simulink model "TosAeb"  (R2021a)
settings: Solver=FixedStepDiscrete  StartTime=0.0  StopTime=inf  FixedStep=0.01  RelTol=1e-3
undefined symbols (not defined in the file or in its folder): ACCModSts, AccSts1, AEB_ObjectMovingOrientation, AEB_ObjectMovingState, CN_BTCActive, CN_CurrentGearR, CN_CurrentGearR_DHT, CN_PTCActive, CN_VDCActive, CN_VSC_EspOff, CN_VSC_FCW_AEB_Error, CN_VSC_FCW_AEB_NoDispay, CN_VSC_FCW_AEB_PerfmDgdtn, CN_VSC_FCW_AEB_TempUnAvlbl, CN_VSC_VehStandstill, CNaccelAvoidMax, CNaccelAvoidMin, CNbrakePedalPress, CNcomfortStrLeftAccelMax, CNcomfortStrLeftAccelMin, CNcomfortStrRightAccelMax, CNcomfortStrRightAccelMin, CNDrvDoorOpen, CNDrvModeEco, CNDrvModeNorm, CNDrvModeOther, CNDrvModeSport, CNDrvSBRreminder, CNNull, CNobjInvalidID, CNPassDoorOpen, CNPassSBRreminder, CNPEBSAccelNegInf, CNPEBSAccelNull, CNPEBSTimeInf, CNPEBSTimeNull, CNSTOPMAX, CNSTOPNULL, CNsubjWidth, CNSwtOFF, CNSwtON, CNTTCMAX, CNTTCNULL, CNVehConfigAEBCycNone, CNVehConfigAEBNone, CNVehConfigAEBPedNone, CNVehConfigFCWNone, CNVehConfigJANone, CollisionSeverity, FALSE, FCWSnvty, JACycObjCalcInfoDefaultParams, JAPedObjCalcInfoDefaultParams, JAVehObjCalcInfoDefaultParams, latCycObjCalcInfoDefaultParams, latPedObjCalcInfoDefaultParams, longObjCalcInfoDefaultParams, ModSts, movingStatus, ObjectType ... (+1112)
libraries: simulink x3031, AEBSFunctionLib x50, Ta_Library x33, AEBSStateMachineLib x23, Mylibrary x12, simulink_extras x7, ObjSelect_lib x3, dspstat3 x2
(part 30 of 102)

== system TosAeb/FcwAebJa_Step_sys/ALG_AEBSystem/ALG_AEBController/AEB_LatObjInfoCalcApp/LatPedObjCalc/LatPedCollisonAssessInfo/ObjIsInvalidJudge ==
  ObjectID : Inport
  Compare To Constant : simulink/Logic and Bit Operations/Compare To Constant  relop===  const=CNobjInvalidID  OutDataTypeStr=boolean
  ObjIsInvalid : Outport
  wires:
  ObjectID/1 -> Compare To Constant/1
  Compare To Constant/1 -> ObjIsInvalid/1

== system TosAeb/FcwAebJa_Step_sys/ALG_AEBSystem/ALG_AEBController/AEB_LatObjInfoCalcApp/LatPedObjCalc/LatPedObjCalc/ObjPreSelectCondCalc ==
  latPedObjInfo : Inport  OutDataTypeStr=Bus: BusAEBLatObj  PortDimensions=1  BusOutputAsStruct=on
  subjVel : Inport  Port=2
  Bus Selector : BusSelector  OutputSignals=ObjectID,dx,dy,ObjectExistProb,dyVariance,ObjectMovingOrientation,ObjectType,ObjectTypeCameraConfirmed,vx
  If : If  IfExpression=u1
  Merge : Merge
  ObjIsInvalidJudge : Subsystem
  TgtPreSelect : Subsystem
  TgtPreSelectDefault : Subsystem  TreatAsAtomicUnit=on
  ObjPreSelectCond : Outport
  wires:
  Merge/1 -> ObjPreSelectCond/1
  Bus Selector/1 -> ObjIsInvalidJudge/1(ObjectID)  "<ObjectID>"
  TgtPreSelectDefault/1(ObjPreSelectCond) -> Merge/1
  TgtPreSelect/1(ObjPreSelectCond) -> Merge/2
  ObjIsInvalidJudge/1(ObjIsInvalid) -> If/1
  If/2 -> TgtPreSelect/Action
  latPedObjInfo/1 -> Bus Selector/1
  If/1 -> TgtPreSelectDefault/Action
  subjVel/1 -> TgtPreSelect/9(subjVel)
  Bus Selector/2 -> TgtPreSelect/1(dx)  "<dx>"
  Bus Selector/3 -> TgtPreSelect/2(dy)  "<dy>"
  Bus Selector/4 -> TgtPreSelect/3(ObjectExistProb)  "<ObjectExistProb>"
  Bus Selector/5 -> TgtPreSelect/4(dyVariance)  "<dyVariance>"
  Bus Selector/6 -> TgtPreSelect/5(ObjectMovingOrientation)  "<ObjectMovingOrientation>"
  Bus Selector/7 -> TgtPreSelect/6(ObjectType)  "<ObjectType>"
  Bus Selector/8 -> TgtPreSelect/7(ObjectTypeCameraConfirmed)  "<ObjectTypeCameraConfirmed>"
  Bus Selector/9 -> TgtPreSelect/8(vx)  "<vx>"

== system TosAeb/FcwAebJa_Step_sys/ALG_AEBSystem/ALG_AEBController/AEB_LatObjInfoCalcApp/LatPedObjCalc/LatPedObjCalc/latPedObjInfoCalc ==
  subjInfo : Inport
  latPedObjInfo : Inport  Port=2  OutDataTypeStr=Bus: BusAEBLatObj
  Action Port : ActionPort  ActionPortLabel=else
  Bus Creator : BusCreator  Inputs=6  OutDataTypeStr=Bus: BusLatPedObjCalcInfo  NonVirtualBus=on
      settings: InheritFromInputs=off
  Bus Creator1 : BusCreator  Inputs=5
  Bus Selector : BusSelector  OutputSignals=dx,vx,ax,vy,ObjectID,ObjectTypeCameraConfirmed,dy,FR_ObjectID,FR_Dx,FR_Dy,FR_Vy,FC_ObjectID,FC_Dx,FC_Dy,FC_Vy
  Bus Selector1 : BusSelector  OutputSignals=subjVel,subjLongAccel,subjYawRate
  ObjColiisionRiskProbCalc : Subsystem
  ObjCrossFlgCal : Subsystem
  SensorTgtInfo2AbsTgtInfoTrans : Subsystem
  Terminator : Terminator
  Terminator1 : Terminator
  latPedCalcInfo : Outport
  wires:
  ObjColiisionRiskProbCalc/2(preCollisonMaxTime) -> Bus Creator/4  "pedCollisionRiskProb_TTC"
  subjInfo/1 -> ObjCrossFlgCal/1(subjInfo), ObjColiisionRiskProbCalc/3(subjInfo), Bus Selector1/1
  Bus Selector/1 -> SensorTgtInfo2AbsTgtInfoTrans/1(dx)  "<dx>"
  Bus Selector/3 -> SensorTgtInfo2AbsTgtInfoTrans/3(ax)  "<ax>"
  [ObjAbsInfo] -> ObjCrossFlgCal/3(ObjAbsInfo)
  SensorTgtInfo2AbsTgtInfoTrans/1(dxFrontBumper) -> Bus Creator1/1  "dxFrontBumper"
  ObjColiisionRiskProbCalc/4(dyCollisionRiskProbOut) -> Terminator1/1
  Bus Selector/2 -> SensorTgtInfo2AbsTgtInfoTrans/2(vx)  "<vx>"
  Bus Selector1/1 -> SensorTgtInfo2AbsTgtInfoTrans/16(subjVel)  "<subjVel>"
  ObjColiisionRiskProbCalc/3(dxCollisionRiskProbOut) -> Terminator/1
  SensorTgtInfo2AbsTgtInfoTrans/2(vxAbsObj) -> Bus Creator1/2  "vxAbsObj"
  Bus Creator1/1 -> ObjColiisionRiskProbCalc/2(ObjAbsInfo), [ObjAbsInfo]
  SensorTgtInfo2AbsTgtInfoTrans/3(axAbsObj) -> Bus Creator1/3  "axAbsObj"
  Bus Selector1/2 -> SensorTgtInfo2AbsTgtInfoTrans/17(subjLongAccel)  "<subjLongAccel>"
  Bus Creator/1 -> latPedCalcInfo/1
  latPedObjInfo/1 -> Bus Creator/1, ObjColiisionRiskProbCalc/1(ObjInfoRaw), Bus Selector/1, ObjCrossFlgCal/2(ObjInfoRaw)  "latPedObjInfo"
  ObjCrossFlgCal/1(pedPreCollisionFlg) -> Bus Creator/5  "pedPreCollisionFlg"
  [ObjAbsInfo] -> Bus Creator/2  "pedObjAbsInfo"
  ObjColiisionRiskProbCalc/1(pedCollisionRiskProb) -> Bus Creator/3  "pedCollisionRiskProb"
  Bus Selector/4 -> SensorTgtInfo2AbsTgtInfoTrans/4(vy)  "<vy>"
  Bus Selector1/3 -> SensorTgtInfo2AbsTgtInfoTrans/18(subjYawRate)  "<subjYawRate>"
  SensorTgtInfo2AbsTgtInfoTrans/4(vyCorr) -> Bus Creator1/4  "vyCorr"
  SensorTgtInfo2AbsTgtInfoTrans/5(ObjFusQuality) -> Bus Creator1/5  "ObjFusQuality"
  Bus Selector/5 -> SensorTgtInfo2AbsTgtInfoTrans/5(ObjectID)  "<ObjectID>"
  Bus Selector/6 -> SensorTgtInfo2AbsTgtInfoTrans/6(ObjectTypeCameraConfirmed)  "<ObjectTypeCameraConfirmed>"
  Bus Selector/8 -> SensorTgtInfo2AbsTgtInfoTrans/8(FR_ObjectID)  "<FR_ObjectID>"
  Bus Selector/11 -> SensorTgtInfo2AbsTgtInfoTrans/11(FR_Vy)  "<FR_Vy>"
  Bus Selector/12 -> SensorTgtInfo2AbsTgtInfoTrans/12(FC_ObjectID)  "<FC_ObjectID>"
  Bus Selector/15 -> SensorTgtInfo2AbsTgtInfoTrans/15(FC_Vy)  "<FC_Vy>"
  Bus Selector/13 -> SensorTgtInfo2AbsTgtInfoTrans/13(FC_Dx)  "<FC_Dx>"
  Bus Selector/9 -> SensorTgtInfo2AbsTgtInfoTrans/9(FR_Dx)  "<FR_Dx>"
  Bus Selector/10 -> SensorTgtInfo2AbsTgtInfoTrans/10(FR_Dy)  "<FR_Dy>"
  Bus Selector/14 -> SensorTgtInfo2AbsTgtInfoTrans/14(FC_Dy)  "<FC_Dy>"
  Bus Selector/7 -> SensorTgtInfo2AbsTgtInfoTrans/7(dy)  "<dy>"
  ObjCrossFlgCal/2(TTC) -> Bus Creator/6  "pedPreCollisionFlg_TTC"

== system TosAeb/FcwAebJa_Step_sys/ALG_AEBSystem/ALG_AEBController/AEB_LatObjInfoCalcApp/LatPedObjCalc/LatPedObjCalc/latPedObjInfoDefault ==
  Action Port : ActionPort  ActionPortLabel=if(u1)
  LatObjCalcInfoDefaultParams : Constant  Value=latPedObjCalcInfoDefaultParams  OutDataTypeStr=Bus: BusLatPedObjCalcInfo
  latPedCalcInfo : Outport
  wires:
  LatObjCalcInfoDefaultParams/1 -> latPedCalcInfo/1

== system TosAeb/FcwAebJa_Step_sys/ALG_AEBSystem/ALG_AEBController/AEB_LatObjInfoCalcApp/LatPedObjCalc/Subsystem/ObjPreSelectCondCalc ==
  latPedObjInfo : Inport  OutDataTypeStr=Bus: BusAEBLatObj  PortDimensions=1  BusOutputAsStruct=on
  subjVel : Inport  Port=2
  Bus Selector : BusSelector  OutputSignals=ObjectID,dx,dy,ObjectExistProb,dyVariance,ObjectMovingOrientation,ObjectType,ObjectTypeCameraConfirmed,vx
  If : If  IfExpression=u1
  Merge : Merge
  ObjIsInvalidJudge : Subsystem
  TgtPreSelect : Subsystem
  TgtPreSelectDefault : Subsystem  TreatAsAtomicUnit=on
  ObjPreSelectCond : Outport
  wires:
  Merge/1 -> ObjPreSelectCond/1
  Bus Selector/1 -> ObjIsInvalidJudge/1(ObjectID)  "<ObjectID>"
  TgtPreSelectDefault/1(ObjPreSelectCond) -> Merge/1
  TgtPreSelect/1(ObjPreSelectCond) -> Merge/2
  ObjIsInvalidJudge/1(ObjIsInvalid) -> If/1
  If/2 -> TgtPreSelect/Action
  latPedObjInfo/1 -> Bus Selector/1
  If/1 -> TgtPreSelectDefault/Action
  subjVel/1 -> TgtPreSelect/9(subjVel)
  Bus Selector/2 -> TgtPreSelect/1(dx)  "<dx>"
  Bus Selector/3 -> TgtPreSelect/2(dy)  "<dy>"
  Bus Selector/4 -> TgtPreSelect/3(ObjectExistProb)  "<ObjectExistProb>"
  Bus Selector/5 -> TgtPreSelect/4(dyVariance)  "<dyVariance>"
  Bus Selector/6 -> TgtPreSelect/5(ObjectMovingOrientation)  "<ObjectMovingOrientation>"
  Bus Selector/7 -> TgtPreSelect/6(ObjectType)  "<ObjectType>"
  Bus Selector/8 -> TgtPreSelect/7(ObjectTypeCameraConfirmed)  "<ObjectTypeCameraConfirmed>"
  Bus Selector/9 -> TgtPreSelect/8(vx)  "<vx>"

== system TosAeb/FcwAebJa_Step_sys/ALG_AEBSystem/ALG_AEBController/AEB_LatObjInfoCalcApp/LatPedObjCalc/Subsystem/latPedObjInfoCalc ==
  subjInfo : Inport
  latPedObjInfo : Inport  Port=2  OutDataTypeStr=Bus: BusAEBLatObj
  Action Port : ActionPort  ActionPortLabel=else
  Bus Creator : BusCreator  Inputs=6  OutDataTypeStr=Bus: BusLatPedObjCalcInfo  NonVirtualBus=on
      settings: InheritFromInputs=off
  Bus Creator1 : BusCreator  Inputs=5
  Bus Selector : BusSelector  OutputSignals=dx,vx,ax,vy,ObjectID,ObjectTypeCameraConfirmed,dy,FR_ObjectID,FR_Dx,FR_Dy,FR_Vy,FC_ObjectID,FC_Dx,FC_Dy,FC_Vy
  Bus Selector1 : BusSelector  OutputSignals=subjVel,subjLongAccel,subjYawRate
  ObjColiisionRiskProbCalc : Subsystem
  ObjCrossFlgCal : Subsystem
  SensorTgtInfo2AbsTgtInfoTrans : Subsystem
  Terminator : Terminator
  Terminator1 : Terminator
  latPedCalcInfo : Outport
  wires:
  ObjColiisionRiskProbCalc/2(preCollisonMaxTime) -> Bus Creator/4  "pedCollisionRiskProb_TTC"
  subjInfo/1 -> ObjCrossFlgCal/1(subjInfo), ObjColiisionRiskProbCalc/3(subjInfo), Bus Selector1/1
  Bus Selector/1 -> SensorTgtInfo2AbsTgtInfoTrans/1(dx)  "<dx>"
  Bus Selector/3 -> SensorTgtInfo2AbsTgtInfoTrans/3(ax)  "<ax>"
  [ObjAbsInfo] -> ObjCrossFlgCal/3(ObjAbsInfo)
  SensorTgtInfo2AbsTgtInfoTrans/1(dxFrontBumper) -> Bus Creator1/1  "dxFrontBumper"
  ObjColiisionRiskProbCalc/4(dyCollisionRiskProbOut) -> Terminator1/1
  Bus Selector/2 -> SensorTgtInfo2AbsTgtInfoTrans/2(vx)  "<vx>"
  Bus Selector1/1 -> SensorTgtInfo2AbsTgtInfoTrans/16(subjVel)  "<subjVel>"
  ObjColiisionRiskProbCalc/3(dxCollisionRiskProbOut) -> Terminator/1
  SensorTgtInfo2AbsTgtInfoTrans/2(vxAbsObj) -> Bus Creator1/2  "vxAbsObj"
  Bus Creator1/1 -> ObjColiisionRiskProbCalc/2(ObjAbsInfo), [ObjAbsInfo]
  SensorTgtInfo2AbsTgtInfoTrans/3(axAbsObj) -> Bus Creator1/3  "axAbsObj"
  Bus Selector1/2 -> SensorTgtInfo2AbsTgtInfoTrans/17(subjLongAccel)  "<subjLongAccel>"
  Bus Creator/1 -> latPedCalcInfo/1
  latPedObjInfo/1 -> Bus Creator/1, ObjColiisionRiskProbCalc/1(ObjInfoRaw), Bus Selector/1, ObjCrossFlgCal/2(ObjInfoRaw)  "latPedObjInfo"
  ObjCrossFlgCal/1(pedPreCollisionFlg) -> Bus Creator/5  "pedPreCollisionFlg"
  [ObjAbsInfo] -> Bus Creator/2  "pedObjAbsInfo"
  ObjColiisionRiskProbCalc/1(pedCollisionRiskProb) -> Bus Creator/3  "pedCollisionRiskProb"
  Bus Selector/4 -> SensorTgtInfo2AbsTgtInfoTrans/4(vy)  "<vy>"
  Bus Selector1/3 -> SensorTgtInfo2AbsTgtInfoTrans/18(subjYawRate)  "<subjYawRate>"
  SensorTgtInfo2AbsTgtInfoTrans/4(vyCorr) -> Bus Creator1/4  "vyCorr"
  SensorTgtInfo2AbsTgtInfoTrans/5(ObjFusQuality) -> Bus Creator1/5  "ObjFusQuality"
  Bus Selector/5 -> SensorTgtInfo2AbsTgtInfoTrans/5(ObjectID)  "<ObjectID>"
  Bus Selector/6 -> SensorTgtInfo2AbsTgtInfoTrans/6(ObjectTypeCameraConfirmed)  "<ObjectTypeCameraConfirmed>"
  Bus Selector/8 -> SensorTgtInfo2AbsTgtInfoTrans/8(FR_ObjectID)  "<FR_ObjectID>"
  Bus Selector/11 -> SensorTgtInfo2AbsTgtInfoTrans/11(FR_Vy)  "<FR_Vy>"
  Bus Selector/12 -> SensorTgtInfo2AbsTgtInfoTrans/12(FC_ObjectID)  "<FC_ObjectID>"
  Bus Selector/15 -> SensorTgtInfo2AbsTgtInfoTrans/15(FC_Vy)  "<FC_Vy>"
  Bus Selector/13 -> SensorTgtInfo2AbsTgtInfoTrans/13(FC_Dx)  "<FC_Dx>"
  Bus Selector/9 -> SensorTgtInfo2AbsTgtInfoTrans/9(FR_Dx)  "<FR_Dx>"
  Bus Selector/10 -> SensorTgtInfo2AbsTgtInfoTrans/10(FR_Dy)  "<FR_Dy>"
  Bus Selector/14 -> SensorTgtInfo2AbsTgtInfoTrans/14(FC_Dy)  "<FC_Dy>"
  Bus Selector/7 -> SensorTgtInfo2AbsTgtInfoTrans/7(dy)  "<dy>"
  ObjCrossFlgCal/2(TTC) -> Bus Creator/6  "pedPreCollisionFlg_TTC"

== system TosAeb/FcwAebJa_Step_sys/ALG_AEBSystem/ALG_AEBController/AEB_LatObjInfoCalcApp/LatPedObjCalc/Subsystem/latPedObjInfoDefault ==
  Action Port : ActionPort  ActionPortLabel=if(u1)
  LatObjCalcInfoDefaultParams : Constant  Value=latPedObjCalcInfoDefaultParams  OutDataTypeStr=Bus: BusLatPedObjCalcInfo
  latPedCalcInfo : Outport
  wires:
  LatObjCalcInfoDefaultParams/1 -> latPedCalcInfo/1

== system TosAeb/FcwAebJa_Step_sys/ALG_AEBSystem/ALG_AEBController/AEB_LongTgtInfoCalc/LongObjCalcInfoCalc/AEBS_LongCollisionAssesApp/DriverReactionTimeCalc ==
  ACCState : Inport  OutDataTypeStr=Enum: ACCModSts
  BrakePedalStatus_G : Inport  Port=2
  ESP_MasterCylindBrakePress : Inport  Port=3
  AccrPedlPosnDiagc : Inport  Port=4
  SteerWheelSpd : Inport  Port=5
  LeftTurnSWSts : Inport  Port=6
  RightTurnSWSts : Inport  Port=7
  FcwHMISetting : Inport  Port=8
  ACCStateMonitor : Subsystem  TreatAsAtomicUnit=on
  DriverStateMonitor : Subsystem  TreatAsAtomicUnit=on
  ReationTimeCalc1 : Subsystem  TreatAsAtomicUnit=on
  Terminator : Terminator
  diverReactionTime : Outport
  wires:
  ESP_MasterCylindBrakePress/1 -> DriverStateMonitor/2(ESP_MasterCylindBrakePress)
  BrakePedalStatus_G/1 -> DriverStateMonitor/1(BrakePedalStatus_G)
  AccrPedlPosnDiagc/1 -> DriverStateMonitor/3(AccrPedlPosnDiagc)
  SteerWheelSpd/1 -> DriverStateMonitor/4(SteerWheelSpd)
  LeftTurnSWSts/1 -> DriverStateMonitor/5(LeftTurnSWSts)
  RightTurnSWSts/1 -> DriverStateMonitor/6(RightTurnSWSts)
  ACCState/1 -> ACCStateMonitor/1(ACCState)
  ACCStateMonitor/2(ACCMonitor) -> ReationTimeCalc1/2(ACCMonitor)
  DriverStateMonitor/1(driverMonitor) -> ReationTimeCalc1/3(driverMonitor)
  ReationTimeCalc1/1(driverReactionTime) -> diverReactionTime/1
  ACCStateMonitor/1(timesincelastACC) -> Terminator/1
  FcwHMISetting/1 -> ReationTimeCalc1/1(FcwHMISetting)

== system TosAeb/FcwAebJa_Step_sys/ALG_AEBSystem/ALG_AEBController/AEB_LongTgtInfoCalc/LongObjCalcInfoCalc/AEBS_LongCollisionAssesApp/LatCollisionAvoidCalc ==
  note: if object is stationary
      | 	Calculate comfort steering based on stationary object;
      | else
      | 	Calculate comfort steering based on moving object;
  TTC : Inport
  subjVel : Inport  Port=2
  ObjectMovingState : Inport  Port=3
  dy : Inport  Port=4
  dxFrontBumper : Inport  Port=5
  vx : Inport  Port=6
  vy : Inport  Port=7
  FcwHMISetting : Inport  Port=8
  AccelLatAvoidCalc4SO : Subsystem
  AccelLatAvoidCalcMO : Subsystem
  Bus Creator1 : BusCreator  Inputs=3
  Bus Selector : BusSelector  OutputSignals=accelLatAvoidLeft,accelLatAvoidRight
  Bus Selector1 : BusSelector  OutputSignals=accelLatAvoidLeft,accelLatAvoidRight
  If : If  IfExpression=u1
  LatAvoidFlg4BrakeJudge : Subsystem
  LatAvoidFlg4WarningJudge : Subsystem
  Merge : Merge
  ObjIsStationaryJudge : Subsystem
  latAvoidAssess : Outport
  wires:
  ObjIsStationaryJudge/1(objIsStationaryFlg) -> If/1
  ObjectMovingState/1 -> [ObjectMovingState], ObjIsStationaryJudge/1(ObjectMovingState)
  LatAvoidFlg4BrakeJudge/1(latAvoidFlg4Brake) -> Bus Creator1/3  "latAvoidFlg4Brake"
  Bus Creator1/1 -> latAvoidAssess/1  "latAvoidAssess"
  LatAvoidFlg4WarningJudge/1(latAvoidFlg4Warning) -> Bus Creator1/2  "latAvoidFlg4Warning"
  FcwHMISetting/1 -> LatAvoidFlg4WarningJudge/2(FcwHMISetting)
  [subjVel] -> LatAvoidFlg4BrakeJudge/3(subjVel)
  Merge/1 -> Bus Selector1/1, Bus Selector/1, Bus Creator1/1  "accelLatAvoid"
  dy/1 -> AccelLatAvoidCalcMO/4(dy), AccelLatAvoidCalc4SO/1(dy)
  [MOVINGOBJ] -> AccelLatAvoidCalcMO/Action
  If/2 -> [MOVINGOBJ]
  If/1 -> AccelLatAvoidCalc4SO/Action
  vy/1 -> AccelLatAvoidCalcMO/5(vy)
  TTC/1 -> AccelLatAvoidCalcMO/3(TTC)
  vx/1 -> AccelLatAvoidCalcMO/2(vx)
  subjVel/1 -> AccelLatAvoidCalc4SO/3(subjVel), [subjVel]
  dxFrontBumper/1 -> AccelLatAvoidCalcMO/1(dxFrontBumper), AccelLatAvoidCalc4SO/2(dxFrontBumper)
  AccelLatAvoidCalcMO/1(accelLatAvoid) -> Merge/2
  AccelLatAvoidCalc4SO/1(accelLatAvoid) -> Merge/1
  [subjVel] -> LatAvoidFlg4WarningJudge/3(subjVel)
  [ObjectMovingState] -> LatAvoidFlg4WarningJudge/1(ObjectMovingState)
  Bus Selector/1 -> LatAvoidFlg4WarningJudge/4(accelLatAvoidLeft)  "<accelLatAvoidLeft>"
  Bus Selector/2 -> LatAvoidFlg4WarningJudge/5(accelLatAvoidRight)  "<accelLatAvoidRight>"
  Bus Selector1/2 -> LatAvoidFlg4BrakeJudge/2(accelLatAvoidRight)  "<accelLatAvoidRight>"
  Bus Selector1/1 -> LatAvoidFlg4BrakeJudge/1(accelLatAvoidLeft)  "<accelLatAvoidLeft>"

== system TosAeb/FcwAebJa_Step_sys/ALG_AEBSystem/ALG_AEBController/AEB_LongTgtInfoCalc/LongObjCalcInfoCalc/AEBS_LongCollisionAssesApp/LongCollisionAvoidAssess ==
  tEgoStop : Inport
  tObjStop : Inport  Port=2
  TTC : Inport  Port=3
  tReaction : Inport  Port=4
  vx : Inport  Port=5
  axAbsObj : Inport  Port=6
  vxAbsObj : Inport  Port=7
  ax : Inport  Port=8
  dxFrontBumper : Inport  Port=9
  subjVel : Inport  Port=10
  subjLongAccel : Inport  Port=11
  FcwHMISetting : Inport  Port=12
  Bus Creator : BusCreator  Inputs=12
  Bus Creator1 : BusCreator  Inputs=7
  Bus Selector : BusSelector  OutputSignals=vx,axAbsObj,vxAbsObj,dxFrontBumper,subjVel,FcwHMISetting,tObjStop
  Bus Selector1 : BusSelector  OutputSignals=tEgoStop,tObjStop,TTC,tReaction,vx,axAbsObj,vxAbsObj,ax,subjLongAccel,dxFrontBumper,subjVel
  HighLvlBrkCalc : Subsystem  TreatAsAtomicUnit=on
  LowLvlBrkCalc : Subsystem  TreatAsAtomicUnit=on
  longCollisionAvoidAssess : Outport
  wires:
  Bus Creator1/1 -> longCollisionAvoidAssess/1  "longCollisionAvoidAssess"
  HighLvlBrkCalc/4(highLvlBrkFlg4Brake) -> Bus Creator1/7  "highLvlBrkFlg4Brake"
  LowLvlBrkCalc/2(lowLvlBrkFlg4Warning) -> Bus Creator1/2  "lowLvlBrkFlg4Warning"
  HighLvlBrkCalc/3(accelAvoidHighLvlBrk4Brake) -> Bus Creator1/6  "accelAvoidHighLvlBrk4Brake"
  LowLvlBrkCalc/3(lowLvlBrkFlg4Brake) -> Bus Creator1/3  "lowLvlBrkFlg4Brake"
  HighLvlBrkCalc/2(highLvlBrkFlg4Warning) -> Bus Creator1/5  "highLvlBrkFlg4Warning"
  HighLvlBrkCalc/1(accelAvoidHighLvlBrk4Waning) -> Bus Creator1/4  "accelAvoidHighLvlBrk4Warning"
  Bus Selector1/1 -> HighLvlBrkCalc/1(tEgoStop)  "<tEgoStop>"
  Bus Selector1/11 -> HighLvlBrkCalc/11(subjVel)  "<subjVel>"
  Bus Selector1/2 -> HighLvlBrkCalc/2(tObjStop)  "<tObjStop>"
  Bus Selector1/10 -> HighLvlBrkCalc/10(dxFrontBumper)  "<dxFrontBumper>"
  Bus Selector1/3 -> HighLvlBrkCalc/3(TTC)  "<TTC>"
  Bus Selector1/9 -> HighLvlBrkCalc/9(subjLongAccel)  "<subjLongAccel>"
  Bus Selector1/4 -> HighLvlBrkCalc/4(tReaction)  "<tReaction>"
  Bus Selector1/8 -> HighLvlBrkCalc/8(ax)  "<ax>"
  Bus Selector1/5 -> HighLvlBrkCalc/5(vx)  "<vx>"
  Bus Selector1/7 -> HighLvlBrkCalc/7(vxAbsObj)  "<vxAbsObj>"
  Bus Selector1/6 -> HighLvlBrkCalc/6(axAbsObj)  "<axAbsObj>"
  FcwHMISetting/1 -> Bus Creator/12  "FcwHMISetting"
  Bus Creator/1 -> Bus Selector/1, Bus Selector1/1
  subjLongAccel/1 -> Bus Creator/11  "subjLongAccel"
  subjVel/1 -> Bus Creator/10  "subjVel"
  dxFrontBumper/1 -> Bus Creator/9  "dxFrontBumper"
  ax/1 -> Bus Creator/8  "ax"
  vxAbsObj/1 -> Bus Creator/7  "vxAbsObj"
  tEgoStop/1 -> Bus Creator/1  "tEgoStop"
  axAbsObj/1 -> Bus Creator/6  "axAbsObj"
  tObjStop/1 -> Bus Creator/2  "tObjStop"
  vx/1 -> Bus Creator/5  "vx"
  TTC/1 -> Bus Creator/3  "TTC"
  tReaction/1 -> Bus Creator/4  "tReaction"
  LowLvlBrkCalc/1(accelAvoidLowLvlBrk) -> Bus Creator1/1  "accelAvoidLowLvlBrk"
  Bus Selector/1 -> LowLvlBrkCalc/1(vx)  "<vx>"
  Bus Selector/2 -> LowLvlBrkCalc/2(axAbsObj)  "<axAbsObj>"
  Bus Selector/3 -> LowLvlBrkCalc/3(vxAbsObj)  "<vxAbsObj>"
  Bus Selector/4 -> LowLvlBrkCalc/4(dxFrontBumper)  "<dxFrontBumper>"
  Bus Selector/5 -> LowLvlBrkCalc/5(subjVel)  "<subjVel>"
  Bus Selector/6 -> LowLvlBrkCalc/6(FcwHMISetting)  "<FcwHMISetting>"
  Bus Selector/7 -> LowLvlBrkCalc/7(tObjStop)  "<tObjStop>"

== system TosAeb/FcwAebJa_Step_sys/ALG_AEBSystem/ALG_AEBController/AEB_LongTgtInfoCalc/LongObjCalcInfoCalc/AEBS_LongCollisionAssesApp/LongCollisionSeverityCalc ==
  collisionSeverityLowFlg : Inport  OutDataTypeStr=boolean
  collisionSeverityMediumFlg : Inport  Port=2  OutDataTypeStr=boolean
  collisionSeverityHighFlg : Inport  Port=3  OutDataTypeStr=boolean
  ObjectID : Inport  Port=4
  LongCollisionSeverityCalcFlow : Stateflow chart  TreatAsAtomicUnit=on
  longCollisionSeverity : Outport
  wires:
  LongCollisionSeverityCalcFlow/1(longCollisionSeverity) -> longCollisionSeverity/1
  collisionSeverityMediumFlg/1 -> LongCollisionSeverityCalcFlow/2(collisionSeverityMediumFlg)  "collisionSeverityMediumFlg"
  collisionSeverityLowFlg/1 -> LongCollisionSeverityCalcFlow/1(collisionSeverityLowFlg)  "collisionSeverityLowFlg"
  collisionSeverityHighFlg/1 -> LongCollisionSeverityCalcFlow/3(collisionSeverityHighFlg)  "collisionSeverityHighFlg"
  ObjectID/1 -> LongCollisionSeverityCalcFlow/4(ObjectID)  "ObjectID"

== system TosAeb/FcwAebJa_Step_sys/ALG_AEBSystem/ALG_AEBController/AEB_LongTgtInfoCalc/LongObjCalcInfoCalc/AEBS_LongCollisionAssesApp/TTCCalc ==
  subjLongAccel : Inport
  subjVel : Inport  Port=2
  dxFrontBumper : Inport  Port=3
  axAbsObj : Inport  Port=4
  vxAbsObj : Inport  Port=5
  ax : Inport  Port=6
  vx : Inport  Port=7
  If : If  IfExpression=u1 <= 0
  Merge : Merge
  TTCCalc : Subsystem  TreatAsAtomicUnit=on
  TTCNull : Subsystem  TreatAsAtomicUnit=on
  TTCCalcOut : Outport
  wires:
  subjVel/1 -> TTCCalc/2(subjVel)
  dxFrontBumper/1 -> If/1, TTCCalc/7(dxFrontBumper)
  subjLongAccel/1 -> TTCCalc/1(subjLongAccel)
  Merge/1 -> TTCCalcOut/1
  vx/1 -> TTCCalc/6(vx)
  TTCCalc/1(TTCCalcOut) -> Merge/2
  If/2 -> TTCCalc/Action
  ax/1 -> TTCCalc/5(ax)
  vxAbsObj/1 -> TTCCalc/4(vxAbsObj)
  If/1 -> TTCNull/Action
  TTCNull/1(TTCCalcOut) -> Merge/1
  axAbsObj/1 -> TTCCalc/3(axAbsObj)

== system TosAeb/FcwAebJa_Step_sys/ALG_AEBSystem/ALG_AEBController/AEB_LongTgtInfoCalc/LongObjCalcInfoCalc/AEBS_LongCollisionAssesApp/collisionSeverityHighCalc ==
  collisionSeverityMediumFlg : Inport
  ObjectMovingState : Inport  Port=2
  vx : Inport  Port=3
  dxFrontBumper : Inport  Port=4
  vxAbsObj : Inport  Port=5
  axAbsObj : Inport  Port=6
  subjLongAccel : Inport  Port=7
  subjVel : Inport  Port=8
  FcwHMISetting : Inport  Port=9
  If : If  IfExpression=~u1
  Merge : Merge
  collisionSeverityHighCalc1 : Subsystem  TreatAsAtomicUnit=on
  collisionSeverityHighCalc2 : Subsystem  Commented=on  TreatAsAtomicUnit=on
  collisionSeverityHighNoFullfilled : Subsystem  TreatAsAtomicUnit=on
  collisionSeverityHighFlg : Outport
  wires:
  If/2 -> collisionSeverityHighCalc1/Action
  If/1 -> collisionSeverityHighNoFullfilled/Action
  collisionSeverityMediumFlg/1 -> If/1
  Merge/1 -> collisionSeverityHighFlg/1  "collisionSeverityHighFlg"
  collisionSeverityHighCalc1/1(collisionSeverityHighFlg) -> Merge/2
  collisionSeverityHighNoFullfilled/1(collisionSeverityHighFlg) -> Merge/1
  ObjectMovingState/1 -> collisionSeverityHighCalc1/1(ObjectMovingState)  "ObjectMovingState"
  vx/1 -> collisionSeverityHighCalc1/7(vx)  "vx"
  dxFrontBumper/1 -> collisionSeverityHighCalc1/6(dxFrontBumper)  "dxFrontBumper"
  vxAbsObj/1 -> collisionSeverityHighCalc1/3(vxAbsObj)  "vxAbsObj"
  axAbsObj/1 -> collisionSeverityHighCalc1/4(axAbsObj)  "axAbsObj"
  subjLongAccel/1 -> collisionSeverityHighCalc1/2(subjLongAccel)  "subjLongAccel"
  subjVel/1 -> collisionSeverityHighCalc1/5(subjVel)  "subjVel"
  FcwHMISetting/1 -> collisionSeverityHighCalc1/8(FCWSnvty)

== system TosAeb/FcwAebJa_Step_sys/ALG_AEBSystem/ALG_AEBController/AEB_LongTgtInfoCalc/LongObjCalcInfoCalc/AEBS_LongCollisionAssesApp/collisionSeverityLowCalc ==
  lowLvlBrkFlg4Warning : Inport
  highLvlBrkFlg4Warning : Inport  Port=2
  latAvoidFlg4Warning : Inport  Port=3
  Compare To Constant : simulink/Logic and Bit Operations/Compare To Constant  relop===  const=FALSE  OutDataTypeStr=boolean
  Compare To Constant1 : simulink/Logic and Bit Operations/Compare To Constant  relop===  const=FALSE  OutDataTypeStr=boolean
  Compare To Constant2 : simulink/Logic and Bit Operations/Compare To Constant  relop===  const=FALSE  OutDataTypeStr=boolean
  Logical Operator2 : Logic  Inputs=3  AllPortsSameDT=off  OutDataTypeStr=boolean
  collisionSeverityLowFlg : Outport
  wires:
  latAvoidFlg4Warning/1 -> Compare To Constant2/1
  Logical Operator2/1 -> collisionSeverityLowFlg/1
  highLvlBrkFlg4Warning/1 -> Compare To Constant1/1
  lowLvlBrkFlg4Warning/1 -> Compare To Constant/1
  Compare To Constant/1 -> Logical Operator2/1
  Compare To Constant1/1 -> Logical Operator2/2
  Compare To Constant2/1 -> Logical Operator2/3

== system TosAeb/FcwAebJa_Step_sys/ALG_AEBSystem/ALG_AEBController/AEB_LongTgtInfoCalc/LongObjCalcInfoCalc/AEBS_LongCollisionAssesApp/collisionSeverityMediumCalc ==
  lowLvlBrkFlg4Brake : Inport
  highLvlBrkFlg4Brake : Inport  Port=2
  latAvoidFlg4Brake : Inport  Port=3
  Compare To Constant : simulink/Logic and Bit Operations/Compare To Constant  relop===  const=FALSE  OutDataTypeStr=boolean
  Compare To Constant1 : simulink/Logic and Bit Operations/Compare To Constant  relop===  const=FALSE  OutDataTypeStr=boolean
  Compare To Constant2 : simulink/Logic and Bit Operations/Compare To Constant  relop===  const=FALSE  OutDataTypeStr=boolean
  Logical Operator2 : Logic  Inputs=3  AllPortsSameDT=off  OutDataTypeStr=boolean
  collisionSeverityMediumFlg : Outport
  wires:
  Compare To Constant2/1 -> Logical Operator2/3
  Compare To Constant1/1 -> Logical Operator2/2
  Compare To Constant/1 -> Logical Operator2/1
  lowLvlBrkFlg4Brake/1 -> Compare To Constant/1
  highLvlBrkFlg4Brake/1 -> Compare To Constant1/1
  Logical Operator2/1 -> collisionSeverityMediumFlg/1
  latAvoidFlg4Brake/1 -> Compare To Constant2/1

== system TosAeb/FcwAebJa_Step_sys/ALG_AEBSystem/ALG_AEBController/AEB_LongTgtInfoCalc/LongObjCalcInfoCalc/ObjAbsInfoTrans/ObjFusQualityCal/SensorThres ==
  subjVel : Inport
  f : TriggerPort  TriggerType=function-call  VariantControl=(inherit)
      settings: CopyFcn=Stateflow.SLINSF.SimulinkMan.removeBlockPreDeleteFcn  PreDeleteFcn=Stateflow.SLINSF.SimulinkMan.unsafePredeleteFcn
  deltaDxThresCalc : Lookup_n-D  NumberOfTableDimensions=1  BreakpointsForDimension1=P_AEB_LongObjAbs_SensorDeltDxThresX  Table=P_AEB_LongObjAbs_SensorDeltDxThresY  ExtrapMethod=Clip  InternalRulePriority=Speed  RndMeth=Simplest  InputSameDT=on
  deltaDyThresCalc : Lookup_n-D  NumberOfTableDimensions=1  BreakpointsForDimension1=P_AEB_LongObjAbs_SensorDeltDyThresX  Table=P_AEB_LongObjAbs_SensorDeltDyThresY  ExtrapMethod=Clip  InternalRulePriority=Speed  RndMeth=Simplest  InputSameDT=on
  deltaFC_FRVxCalc : Lookup_n-D  NumberOfTableDimensions=1  BreakpointsForDimension1=P_AEB_LongObjAbs_deltaFC_FRVxThresX  Table=P_AEB_LongObjAbs_deltaFC_FRVxThresY  ExtrapMethod=Clip  InternalRulePriority=Speed  RndMeth=Simplest  InputSameDT=on
  deltaFC_FusVxCalc : Lookup_n-D  NumberOfTableDimensions=1  BreakpointsForDimension1=P_AEB_LongObjAbs_deltaFC_FusVxThresX  Table=P_AEB_LongObjAbs_deltaFC_FusVxThresY  ExtrapMethod=Clip  InternalRulePriority=Speed  RndMeth=Simplest  InputSameDT=on
  deltaFR_FusVxCalc : Lookup_n-D  NumberOfTableDimensions=1  BreakpointsForDimension1=P_AEB_LongObjAbs_deltaFR_FusVxThresX  Table=P_AEB_LongObjAbs_deltaFR_FusVxThresY  ExtrapMethod=Clip  InternalRulePriority=Speed  RndMeth=Simplest  InputSameDT=on
  deltaDxThres : Outport  OutDataTypeStr=single
  deltaDyThres : Outport  Port=2  OutDataTypeStr=single
  deltaFC_FRVxThres : Outport  Port=3
  deltaFR_FusVxThres : Outport  Port=4
  deltaFC_FusVxThres : Outport  Port=5
  wires:
  deltaDxThresCalc/1 -> deltaDxThres/1
  deltaDyThresCalc/1 -> deltaDyThres/1
  subjVel/1 -> deltaDxThresCalc/1, deltaFC_FRVxCalc/1, deltaFR_FusVxCalc/1, deltaFC_FusVxCalc/1, deltaDyThresCalc/1
  deltaFC_FusVxCalc/1 -> deltaFC_FusVxThres/1
  deltaFC_FRVxCalc/1 -> deltaFC_FRVxThres/1
  deltaFR_FusVxCalc/1 -> deltaFR_FusVxThres/1

== system TosAeb/FcwAebJa_Step_sys/ALG_AEBSystem/ALG_AEBController/AEB_LongTgtInfoCalc/LongObjCalcInfoCalc/OverlapCalculation/OverlapRatioCalcFlow/overlapRatioCalc ==
  note: dyCriticalEgoObj - Abs(dyOverlapActual)
      | overlapRatio = -------------------------------------------------------------
      | 						dyCriticalEgoObj
  dyCriticalEgoObj : Inport
  dyOverlapActual : Inport  Port=2
  f : TriggerPort  TriggerType=function-call  VariantControl=Variant
      settings: CopyFcn=Stateflow.SLINSF.SimulinkMan.removeBlockPreDeleteFcn  PreDeleteFcn=Stateflow.SLINSF.SimulinkMan.unsafePredeleteFcn
  Abs2 : Abs
  Add1 : Sum  Inputs=+-
  Divide : Product  Inputs=*/
  overlapRatio : Outport
  wires:
  Add1/1 -> Divide/1
  Abs2/1 -> Add1/2
  Divide/1 -> overlapRatio/1
  dyCriticalEgoObj/1 -> Add1/1, Divide/2
  dyOverlapActual/1 -> Abs2/1

== system TosAeb/FcwAebJa_Step_sys/ALG_AEBSystem/ALG_AEBController/AEB_LongTgtInfoCalc/LongObjCalcInfoCalc/OverlapCalculation/TargetWidthCalc ==
  ObjectWidth : Inport
  ObjectTypeCameraConfirmed : Inport  Port=2
  ObjectMovingState : Inport  Port=3
  Compare To Constant2 : simulink/Logic and Bit Operations/Compare To Constant  relop===  const=TRUE  OutDataTypeStr=boolean
  MovingObjWidth : Constant  Value=P_AEB_LongCA_OverlapEgoWidthMO
  StationaryObjWidth : Constant  Value=P_AEB_LongCA_OverlapEgoWidthSO
  Switch : Switch  Criteria=u2 ~= 0
  Switch1 : Switch  Criteria=u2 ~= 0
  TargetIsStanding : simulink/Logic and Bit Operations/Compare To Constant  relop===  const=AEB_ObjectMovingState.Still  OutDataTypeStr=boolean
  objWidth : Outport
  wires:
  MovingObjWidth/1 -> Switch1/3
  TargetIsStanding/1 -> Switch1/2
  ObjectTypeCameraConfirmed/1 -> Compare To Constant2/1
  Compare To Constant2/1 -> Switch/2
  Switch1/1 -> Switch/3
  ObjectWidth/1 -> Switch/1
  StationaryObjWidth/1 -> Switch1/1
  ObjectMovingState/1 -> TargetIsStanding/1
  Switch/1 -> objWidth/1

== system TosAeb/FcwAebJa_Step_sys/ALG_AEBSystem/ALG_AEBController/AEB_LongTgtInfoCalc/LongObjCalcInfoCalc/OverlapCalculation/dyCriticalEgoObjCalc ==
  objWidth : Inport
  Add : Sum
  Constant : Constant  Value=P_Global_VehicleWidthMin_m
  Gain1 : Gain  Gain=0.5
  Gain2 : Gain  Gain=0.5
  dyCriticalEgoObj : Outport
  wires:
  objWidth/1 -> Gain1/1
  Constant/1 -> Gain2/1
  Add/1 -> dyCriticalEgoObj/1  "dyCriticalEgoObj"
  Gain1/1 -> Add/1
  Gain2/1 -> Add/2

== system TosAeb/FcwAebJa_Step_sys/ALG_AEBSystem/ALG_AEBController/AEB_LongTgtInfoCalc/LongObjCalcInfoCalc/OverlapCalculation/dyOverlapActualCalc1 ==
  dyEgo2Center : Inport
  dyObject2Center : Inport  Port=2
  Subtract : Sum  Inputs=+-
  dyOverlapActual : Outport
  wires:
  dyObject2Center/1 -> Subtract/2  "dyObject2Center"
  dyEgo2Center/1 -> Subtract/1  "dyEgo2Center"
  Subtract/1 -> dyOverlapActual/1  "dyOverlap"

== system TosAeb/FcwAebJa_Step_sys/ALG_AEBSystem/ALG_AEBController/AEB_LongTgtInfoCalc/LongObjCalcInfoCalc/ProbEscapeCalcu/MovingObjdyEscapeCalcu ==
  subjVel : Inport
  dy : Inport  Port=2
  vx : Inport  Port=3
  vy : Inport  Port=4
  subjYawRate : Inport  Port=5
  subjCurvTraj : Inport  Port=6
  dxFrontBumper : Inport  Port=7
  TTC : Inport  Port=8
  ObjIsMoving : ActionPort  ActionPortLabel=else
  Bus Creator1 : BusCreator  Inputs=2
  RadicalRelevantCalc : Subsystem
  RadicalToXYTrans : Subsystem
  XYToRadicalTrans : Subsystem
  dyEscapeFinalCalc : Subsystem
  sincosCoefCalc : Subsystem
  vyAbsTargetFinalCalc : Subsystem
  dyEscape : Outport
  wires:
  sincosCoefCalc/1(sincosCoef) -> XYToRadicalTrans/3(sincosCoef), RadicalToXYTrans/3(sincosCoef)
  vx/1 -> Bus Creator1/1  "vx"
  vy/1 -> Bus Creator1/2  "vy"
  Bus Creator1/1 -> XYToRadicalTrans/1(LongLatVelRel)  "LongLatVelRel"
  dy/1 -> [dyTargetObjCalc], RadicalRelevantCalc/1(dy)
  dxFrontBumper/1 -> [dxFrontBumper], RadicalRelevantCalc/2(dxFrontBumper)
  subjYawRate/1 -> [subjYawRate], RadicalRelevantCalc/3(subjYawRate)
  subjVel/1 -> RadicalToXYTrans/1(subjVel)
  XYToRadicalTrans/1(velRadicalXY) -> RadicalToXYTrans/2(velRadicalXY)
  TTC/1 -> vyAbsTargetFinalCalc/3(TTC), dyEscapeFinalCalc/5(TTC)
  RadicalToXYTrans/1(velAbsTargetCorr) -> vyAbsTargetFinalCalc/1(velAbsTargetCorr)
  RadicalRelevantCalc/2(objAngleToRear) -> sincosCoefCalc/1(angle)  "objAngleToRear"
  RadicalRelevantCalc/1(radialVelRel) -> XYToRadicalTrans/2(radialVelRel)  "radialVelRel"
  subjCurvTraj/1 -> dyEscapeFinalCalc/3("subjCurvTraj ")
  vyAbsTargetFinalCalc/1(vyAbsTargetFinal) -> dyEscapeFinalCalc/4(vyAbsTargetFinal)
  dyEscapeFinalCalc/1(dyEscape) -> dyEscape/1  "dyEscape"
  [dyTargetObjCalc] -> dyEscapeFinalCalc/1(dy)
  [dxFrontBumper] -> dyEscapeFinalCalc/2(dxFrontBumper)
  [subjYawRate] -> vyAbsTargetFinalCalc/2(subjYawRate)

== system TosAeb/FcwAebJa_Step_sys/ALG_AEBSystem/ALG_AEBController/AEB_LongTgtInfoCalc/LongObjCalcInfoCalc/ProbEscapeCalcu/ProbEscapeCalc ==
  ObjWidth : Inport
  dyEscape : Inport  Port=2
  Abs : Abs
  Add : Sum
  Constant : Constant  OutDataTypeStr=single
  Constant1 : Constant  Value=P_Global_VehicleWidthMin_m
  Divide : Product  Inputs=*/
  Gain : Gain  Gain=0.5
  Gain1 : Gain  Gain=0.5
  Min : MinMax  Inputs=2
  probEscape : Outport
  wires:
  Constant/1 -> Min/2
  Min/1 -> probEscape/1
  Divide/1 -> Min/1
  ObjWidth/1 -> Gain1/1
  Gain/1 -> Add/2
  Gain1/1 -> Add/1
  dyEscape/1 -> Abs/1
  Abs/1 -> Divide/1
  Add/1 -> Divide/2  "dyCriticalEgoObj"
  Constant1/1 -> Gain/1

== system TosAeb/FcwAebJa_Step_sys/ALG_AEBSystem/ALG_AEBController/AEB_LongTgtInfoCalc/LongObjCalcInfoCalc/ProbEscapeCalcu/StationaryObjEscapeCalcu ==
  dy : Inport
  subjLatAccel : Inport  Port=2
  TTC : Inport  Port=3
  ObjIsStationary : ActionPort  ActionPortLabel=if(u1)
  Gain : Gain  Gain=0.5
  Math Function1 : Math  Operator=square  SignedPower=on  SaturateOnIntegerOverflow=on
  Product1 : Product
  Subtract : Sum  Inputs=+-
  dyEscape : Outport
  wires:
  Math Function1/1 -> Product1/2
  dy/1 -> Subtract/1
  Subtract/1 -> dyEscape/1
  subjLatAccel/1 -> Product1/1
  TTC/1 -> Math Function1/1
  Product1/1 -> Gain/1
  Gain/1 -> Subtract/2

== system TosAeb/FcwAebJa_Step_sys/ALG_AEBSystem/ALG_AEBController/AEB_LongTgtInfoCalc/LongObjCalcInfoCalc/ProbEscapeCalcu/Subsystem ==
  dxFrontBumper : Inport
  subjVel : Inport  Port=2
  If : If  IfExpression=u1
  Merge : Merge
  TimegapCalc : Subsystem  TreatAsAtomicUnit=on
  TimegapCalcDefault : Subsystem  TreatAsAtomicUnit=on
  subjVelZeroFlgCalc : Subsystem
  Timegap : Outport
  wires:
  TimegapCalcDefault/1(TTC) -> Merge/1
  Merge/1 -> Timegap/1  "Timegap"
  subjVel/1 -> TimegapCalc/2(vx), subjVelZeroFlgCalc/1(subjVel)
  TimegapCalc/1(TTC) -> Merge/2
  If/1 -> TimegapCalcDefault/Action
  If/2 -> TimegapCalc/Action
  subjVelZeroFlgCalc/1(subjVelZeroFlg) -> If/1
  dxFrontBumper/1 -> TimegapCalc/1(dxFrontBumper)

== system TosAeb/FcwAebJa_Step_sys/ALG_AEBSystem/ALG_AEBController/AEB_LongTgtInfoCalc/LongObjCalcInfoCalc/ProbEscapeCalcu/TargetIsStandingConfirm ==
  ObjectMovingState : Inport
  TargetIsStanding : simulink/Logic and Bit Operations/Compare To Constant  relop===  const=AEB_ObjectMovingState.Still  OutDataTypeStr=boolean
  TargetIsStandingFlg : Outport
  wires:
  ObjectMovingState/1 -> TargetIsStanding/1
  TargetIsStanding/1 -> TargetIsStandingFlg/1

== system TosAeb/FcwAebJa_Step_sys/ALG_AEBSystem/ALG_AEBController/AEB_LongTgtInfoCalc/LongObjCalcInfoCalc/ProbEscapeCalcu/TargetWidthCalc ==
  ObjectWidth : Inport
  ObjectTypeCameraConfirmed : Inport  Port=2
  ObjectMovingState : Inport  Port=3
  Compare To Constant2 : simulink/Logic and Bit Operations/Compare To Constant  relop===  const=TRUE  OutDataTypeStr=boolean
  MovingObjWidth : Constant  Value=P_AEB_LongCA_EscapeEgoWidthSO
  StationaryObjWidth : Constant  Value=P_AEB_LongCA_EscapeEgoWidthSO
  Switch : Switch  Criteria=u2 ~= 0
  Switch1 : Switch  Criteria=u2 ~= 0
  TargetIsStanding : simulink/Logic and Bit Operations/Compare To Constant  relop===  const=AEB_ObjectMovingState.Still  OutDataTypeStr=boolean
  ObjWidth : Outport
  wires:
  MovingObjWidth/1 -> Switch1/3
  TargetIsStanding/1 -> Switch1/2
  ObjectTypeCameraConfirmed/1 -> Compare To Constant2/1
  Compare To Constant2/1 -> Switch/2
  Switch1/1 -> Switch/3
  ObjectWidth/1 -> Switch/1
  StationaryObjWidth/1 -> Switch1/1
  ObjectMovingState/1 -> TargetIsStanding/1
  Switch/1 -> ObjWidth/1

== system TosAeb/FcwAebJa_Step_sys/ALG_AEBSystem/ALG_AEBController/AEB_StateMachineCalcApp/JA_AEBStateMachine/JA_PTW_AEBfullStateMachine/AEBFuncFaultMap ==
  FCW_AEB_VehFctSts : Inport
  Compare To Constant : simulink/Logic and Bit Operations/Compare To Constant  relop===  const=CN_VSC_FCW_AEB_Error  OutDataTypeStr=boolean
  Fault : Outport
  wires:
  FCW_AEB_VehFctSts/1 -> Compare To Constant/1
  Compare To Constant/1 -> Fault/1

== system TosAeb/FcwAebJa_Step_sys/ALG_AEBSystem/ALG_AEBController/AEB_StateMachineCalcApp/JA_AEBStateMachine/JA_PTW_AEBfullStateMachine/ActivationCondCalc ==
  JA_PTW_AEBfullSuppCond : Inport
  JA_PTW_AEBfullSuppArb : Inport  Port=2
  PTW_AEBfullFuncImpSuppCond : Inport  Port=3
  Compare To Constant1 : simulink/Logic and Bit Operations/Compare To Constant  relop===  const=FALSE  OutDataTypeStr=boolean
  Compare To Constant2 : simulink/Logic and Bit Operations/Compare To Constant  relop===  const=FALSE  OutDataTypeStr=boolean
  Compare To Constant3 : simulink/Logic and Bit Operations/Compare To Constant  relop===  const=FALSE  OutDataTypeStr=boolean
  Logical Operator : Logic  Inputs=3  AllPortsSameDT=off  OutDataTypeStr=boolean
  ActivationCondFulfilled : Outport
  wires:
  JA_PTW_AEBfullSuppCond/1 -> Compare To Constant3/1
  JA_PTW_AEBfullSuppArb/1 -> Compare To Constant1/1
  PTW_AEBfullFuncImpSuppCond/1 -> Compare To Constant2/1
  Compare To Constant1/1 -> Logical Operator/2
  Compare To Constant3/1 -> Logical Operator/1
  Compare To Constant2/1 -> Logical Operator/3
  Logical Operator/1 -> ActivationCondFulfilled/1

== system TosAeb/FcwAebJa_Step_sys/ALG_AEBSystem/ALG_AEBController/AEB_StateMachineCalcApp/JA_AEBStateMachine/JA_PTW_AEBfullStateMachine/ActiveStateAborCondCalc ==
  JA_PTW_AEBfullAborCond : Inport
  JA_PTW_AEBfullAborArb : Inport  Port=2
  JA_PTW_AEBfullFuncImpAborCond : Inport  Port=3
  JABrkManeuAborCond : Inport  Port=4
  Compare To Constant{,1..3} (4 alike) : simulink/Logic and Bit Operations/Compare To Constant  relop===  const=TRUE  OutDataTypeStr=boolean
  Logical Operator : Logic  Operator=OR  Inputs=4  AllPortsSameDT=off  OutDataTypeStr=boolean
  ActiveStateAborCondFulfilled : Outport
  wires:
  Logical Operator/1 -> ActiveStateAborCondFulfilled/1
  Compare To Constant2/1 -> Logical Operator/3
  Compare To Constant/1 -> Logical Operator/1
  Compare To Constant1/1 -> Logical Operator/2
  JA_PTW_AEBfullFuncImpAborCond/1 -> Compare To Constant2/1
  JA_PTW_AEBfullAborArb/1 -> Compare To Constant1/1
  JA_PTW_AEBfullAborCond/1 -> Compare To Constant/1
  JABrkManeuAborCond/1 -> Compare To Constant3/1
  Compare To Constant3/1 -> Logical Operator/4

== system TosAeb/FcwAebJa_Step_sys/ALG_AEBSystem/ALG_AEBController/AEB_StateMachineCalcApp/JA_AEBStateMachine/JA_PTW_AEBfullStateMachine/StandstillStateAborCondCalc ==
  JA_PTW_AEBfullAborCond : Inport
  JA_PTW_AEBfullAborArb : Inport  Port=2
  JA_PTW_AEBfullFuncImpAborCond : Inport  Port=3
  JABrkManeuAborCond : Inport  Port=4
  Compare To Constant{,1..3} (4 alike) : simulink/Logic and Bit Operations/Compare To Constant  relop===  const=TRUE  OutDataTypeStr=boolean
  Logical Operator : Logic  Operator=OR  Inputs=4  AllPortsSameDT=off  OutDataTypeStr=boolean
  ActiveStateAborCondFulfilled : Outport
  wires:
  Compare To Constant3/1 -> Logical Operator/4
  JABrkManeuAborCond/1 -> Compare To Constant3/1
  JA_PTW_AEBfullAborCond/1 -> Compare To Constant/1
  JA_PTW_AEBfullAborArb/1 -> Compare To Constant1/1
  JA_PTW_AEBfullFuncImpAborCond/1 -> Compare To Constant2/1
  Compare To Constant1/1 -> Logical Operator/2
  Compare To Constant/1 -> Logical Operator/1
  Compare To Constant2/1 -> Logical Operator/3
  Logical Operator/1 -> ActiveStateAborCondFulfilled/1

== system TosAeb/FcwAebJa_Step_sys/ALG_AEBSystem/ALG_AEBController/AEB_StateMachineCalcApp/JA_AEBStateMachine/JA_PTW_PrefillStateMachine/AEBFuncFaultMap ==
  FCW_AEB_VehFctSts : Inport
  Compare To Constant : simulink/Logic and Bit Operations/Compare To Constant  relop===  const=CN_VSC_FCW_AEB_Error  OutDataTypeStr=boolean
  Fault : Outport
  wires:
  FCW_AEB_VehFctSts/1 -> Compare To Constant/1
  Compare To Constant/1 -> Fault/1

== system TosAeb/FcwAebJa_Step_sys/ALG_AEBSystem/ALG_AEBController/AEB_StateMachineCalcApp/JA_AEBStateMachine/JA_PTW_PrefillStateMachine/ActivationCondCalc ==
  JA_PTW_PrefillSuppCond : Inport
  JA_PTW_PrefillSuppArb : Inport  Port=2
  PTW_PrefillFuncImpSuppCond : Inport  Port=3
  Compare To Constant1 : simulink/Logic and Bit Operations/Compare To Constant  relop===  const=FALSE  OutDataTypeStr=boolean
  Compare To Constant2 : simulink/Logic and Bit Operations/Compare To Constant  relop===  const=FALSE  OutDataTypeStr=boolean
  Compare To Constant3 : simulink/Logic and Bit Operations/Compare To Constant  relop===  const=FALSE  OutDataTypeStr=boolean
  Logical Operator : Logic  Inputs=3  AllPortsSameDT=off  OutDataTypeStr=boolean
  ActivationCondFulfilled : Outport
  wires:
  JA_PTW_PrefillSuppCond/1 -> Compare To Constant3/1
  JA_PTW_PrefillSuppArb/1 -> Compare To Constant1/1
  PTW_PrefillFuncImpSuppCond/1 -> Compare To Constant2/1
  Compare To Constant1/1 -> Logical Operator/2
  Compare To Constant3/1 -> Logical Operator/1
  Compare To Constant2/1 -> Logical Operator/3
  Logical Operator/1 -> ActivationCondFulfilled/1

== system TosAeb/FcwAebJa_Step_sys/ALG_AEBSystem/ALG_AEBController/AEB_StateMachineCalcApp/JA_AEBStateMachine/JA_PTW_PrefillStateMachine/ActiveStateAborCondCalc ==
  JA_PTW_PrefillAborCond : Inport
  JA_PTW_PrefillAborArb : Inport  Port=2
  PTW_PrefillFuncImpAborCond : Inport  Port=3
  ABPManeuAborCond : Inport  Port=4
  Compare To Constant{,1..3} (4 alike) : simulink/Logic and Bit Operations/Compare To Constant  relop===  const=TRUE  OutDataTypeStr=boolean
  Logical Operator : Logic  Operator=OR  Inputs=4  AllPortsSameDT=off  OutDataTypeStr=boolean
  ActiveStateAborCondFulfilled : Outport
  wires:
  Logical Operator/1 -> ActiveStateAborCondFulfilled/1
  Compare To Constant2/1 -> Logical Operator/3
  Compare To Constant/1 -> Logical Operator/1
  Compare To Constant1/1 -> Logical Operator/2
  PTW_PrefillFuncImpAborCond/1 -> Compare To Constant2/1
  JA_PTW_PrefillAborArb/1 -> Compare To Constant1/1
  JA_PTW_PrefillAborCond/1 -> Compare To Constant/1
  ABPManeuAborCond/1 -> Compare To Constant3/1
  Compare To Constant3/1 -> Logical Operator/4

== system TosAeb/FcwAebJa_Step_sys/ALG_AEBSystem/ALG_AEBController/AEB_StateMachineCalcApp/JA_AEBStateMachine/JA_cycAEBfullStateMachine/AEBFuncFaultMap ==
  FCW_AEB_VehFctSts : Inport
  Compare To Constant : simulink/Logic and Bit Operations/Compare To Constant  relop===  const=CN_VSC_FCW_AEB_Error  OutDataTypeStr=boolean
  Fault : Outport
  wires:
  Compare To Constant/1 -> Fault/1
  FCW_AEB_VehFctSts/1 -> Compare To Constant/1

== system TosAeb/FcwAebJa_Step_sys/ALG_AEBSystem/ALG_AEBController/AEB_StateMachineCalcApp/JA_AEBStateMachine/JA_cycAEBfullStateMachine/ActivationCondCalc ==
  JA_cycAEBfullSuppCond : Inport
  JA_cycAEBfullSuppArb : Inport  Port=2
  JA_cycFuncImpSuppCond : Inport  Port=3
  Compare To Constant1 : simulink/Logic and Bit Operations/Compare To Constant  relop===  const=FALSE  OutDataTypeStr=boolean
  Compare To Constant2 : simulink/Logic and Bit Operations/Compare To Constant  relop===  const=FALSE  OutDataTypeStr=boolean
  Compare To Constant3 : simulink/Logic and Bit Operations/Compare To Constant  relop===  const=FALSE  OutDataTypeStr=boolean
  Logical Operator : Logic  Inputs=3  AllPortsSameDT=off  OutDataTypeStr=boolean
  ActivationCondFulfilled : Outport
  wires:
  Logical Operator/1 -> ActivationCondFulfilled/1
  Compare To Constant2/1 -> Logical Operator/3
  Compare To Constant3/1 -> Logical Operator/1
  Compare To Constant1/1 -> Logical Operator/2
  JA_cycFuncImpSuppCond/1 -> Compare To Constant2/1
  JA_cycAEBfullSuppArb/1 -> Compare To Constant1/1
  JA_cycAEBfullSuppCond/1 -> Compare To Constant3/1

== system TosAeb/FcwAebJa_Step_sys/ALG_AEBSystem/ALG_AEBController/AEB_StateMachineCalcApp/JA_AEBStateMachine/JA_cycAEBfullStateMachine/ActiveStateAborCondCalc ==
  JA_cycAEBfullAborCond : Inport
  JA_cycAEBfullAborArb : Inport  Port=2
  JA_cycAEBfullFuncImpAborCond : Inport  Port=3
  JABrkManeuAborCond : Inport  Port=4
  Compare To Constant{,1..3} (4 alike) : simulink/Logic and Bit Operations/Compare To Constant  relop===  const=TRUE  OutDataTypeStr=boolean
  Logical Operator : Logic  Operator=OR  Inputs=4  AllPortsSameDT=off  OutDataTypeStr=boolean
  ActiveStateAborCondFulfilled : Outport
  wires:
  Compare To Constant3/1 -> Logical Operator/4
  JABrkManeuAborCond/1 -> Compare To Constant3/1
  JA_cycAEBfullAborCond/1 -> Compare To Constant/1
  JA_cycAEBfullAborArb/1 -> Compare To Constant1/1
  JA_cycAEBfullFuncImpAborCond/1 -> Compare To Constant2/1
  Compare To Constant1/1 -> Logical Operator/2
  Compare To Constant/1 -> Logical Operator/1
  Compare To Constant2/1 -> Logical Operator/3
  Logical Operator/1 -> ActiveStateAborCondFulfilled/1

== system TosAeb/FcwAebJa_Step_sys/ALG_AEBSystem/ALG_AEBController/AEB_StateMachineCalcApp/JA_AEBStateMachine/JA_cycAEBfullStateMachine/StandstillStateAborCondCalc ==
  JA_cycAEBfullAborCond : Inport
  JA_cycAEBfullAborArb : Inport  Port=2
  JA_cycAEBfullFuncImpAborCond : Inport  Port=3
  JABrkManeuAborCond : Inport  Port=4
  Compare To Constant{,1..3} (4 alike) : simulink/Logic and Bit Operations/Compare To Constant  relop===  const=TRUE  OutDataTypeStr=boolean
  Logical Operator : Logic  Operator=OR  Inputs=4  AllPortsSameDT=off  OutDataTypeStr=boolean
  ActiveStateAborCondFulfilled : Outport
  wires:
  Logical Operator/1 -> ActiveStateAborCondFulfilled/1
  Compare To Constant2/1 -> Logical Operator/3
  Compare To Constant/1 -> Logical Operator/1
  Compare To Constant1/1 -> Logical Operator/2
  JA_cycAEBfullFuncImpAborCond/1 -> Compare To Constant2/1
  JA_cycAEBfullAborArb/1 -> Compare To Constant1/1
  JA_cycAEBfullAborCond/1 -> Compare To Constant/1
  JABrkManeuAborCond/1 -> Compare To Constant3/1
  Compare To Constant3/1 -> Logical Operator/4

== system TosAeb/FcwAebJa_Step_sys/ALG_AEBSystem/ALG_AEBController/AEB_StateMachineCalcApp/JA_AEBStateMachine/JA_cycPrefillStateMachine/AEBFuncFaultMap ==
  FCW_AEB_VehFctSts : Inport
  Compare To Constant : simulink/Logic and Bit Operations/Compare To Constant  relop===  const=CN_VSC_FCW_AEB_Error  OutDataTypeStr=boolean
  Fault : Outport
  wires:
  Compare To Constant/1 -> Fault/1
  FCW_AEB_VehFctSts/1 -> Compare To Constant/1

== system TosAeb/FcwAebJa_Step_sys/ALG_AEBSystem/ALG_AEBController/AEB_StateMachineCalcApp/JA_AEBStateMachine/JA_cycPrefillStateMachine/ActivationCondCalc ==
  JA_cycPrefillSuppCond : Inport
  JA_cycPrefillSuppArb : Inport  Port=2
  JA_cycPrefillFuncImpSuppCond : Inport  Port=3
  Compare To Constant1 : simulink/Logic and Bit Operations/Compare To Constant  relop===  const=FALSE  OutDataTypeStr=boolean
  Compare To Constant2 : simulink/Logic and Bit Operations/Compare To Constant  relop===  const=FALSE  OutDataTypeStr=boolean
  Compare To Constant3 : simulink/Logic and Bit Operations/Compare To Constant  relop===  const=FALSE  OutDataTypeStr=boolean
  Logical Operator : Logic  Inputs=3  AllPortsSameDT=off  OutDataTypeStr=boolean
  ActivationCondFulfilled : Outport
  wires:
  Logical Operator/1 -> ActivationCondFulfilled/1
  Compare To Constant2/1 -> Logical Operator/3
  Compare To Constant3/1 -> Logical Operator/1
  Compare To Constant1/1 -> Logical Operator/2
  JA_cycPrefillFuncImpSuppCond/1 -> Compare To Constant2/1
  JA_cycPrefillSuppArb/1 -> Compare To Constant1/1
  JA_cycPrefillSuppCond/1 -> Compare To Constant3/1

== system TosAeb/FcwAebJa_Step_sys/ALG_AEBSystem/ALG_AEBController/AEB_StateMachineCalcApp/JA_AEBStateMachine/JA_cycPrefillStateMachine/ActiveStateAborCondCalc ==
  JA_cycPrefillAborCond : Inport
  JA_cycPrefillAborArb : Inport  Port=2
  JA_cycFuncImpAborCond : Inport  Port=3
  ABPManeuAborCond : Inport  Port=4
  Compare To Constant{,1..3} (4 alike) : simulink/Logic and Bit Operations/Compare To Constant  relop===  const=TRUE  OutDataTypeStr=boolean
  Logical Operator : Logic  Operator=OR  Inputs=4  AllPortsSameDT=off  OutDataTypeStr=boolean
  ActiveStateAborCondFulfilled : Outport
  wires:
  Compare To Constant3/1 -> Logical Operator/4
  ABPManeuAborCond/1 -> Compare To Constant3/1
  JA_cycPrefillAborCond/1 -> Compare To Constant/1
  JA_cycPrefillAborArb/1 -> Compare To Constant1/1
  JA_cycFuncImpAborCond/1 -> Compare To Constant2/1
  Compare To Constant1/1 -> Logical Operator/2
  Compare To Constant/1 -> Logical Operator/1
  Compare To Constant2/1 -> Logical Operator/3
  Logical Operator/1 -> ActiveStateAborCondFulfilled/1

== system TosAeb/FcwAebJa_Step_sys/ALG_AEBSystem/ALG_AEBController/AEB_StateMachineCalcApp/JA_AEBStateMachine/JA_pedAEBfullStateMachine/AEBFuncFaultMap ==
  FCW_AEB_VehFctSts : Inport
  Compare To Constant : simulink/Logic and Bit Operations/Compare To Constant  relop===  const=CN_VSC_FCW_AEB_Error  OutDataTypeStr=boolean
  Fault : Outport
  wires:
  FCW_AEB_VehFctSts/1 -> Compare To Constant/1
  Compare To Constant/1 -> Fault/1
